simulink model "Complete_Model"  (R2023a)
settings: Solver=VariableStepAuto  StartTime=0.0  StopTime=22  MinStep=5e-5  RelTol=1e-3
values from neighbouring files (symbols this model uses):
  Cbus = 80e-3*n_wt_agg*u^2    (= 1.33333333333)    [param.m]
  D = 1e-3    (= 0.001)    [param.m]
  f = 50    [param.m]
  Fs = Vs * sqrt(2/3)/(2*pi*f)    [param.m]
  fsw = 4e3    (= 4000)    [param.m]
  Idr_rated = sqrt(2)*Is    [param.m]
  J = 127*n_wt_agg    (= 19050)    [param.m]
  ki_id = (wni^2)*Lr*sigma    (= 3.27733001332)    [param.m]
  ki_idg = (wnig^2)*Lg    (= 0.263189450696)    [param.m]
  ki_iq = ki_id    (= 3.27733001332)    [param.m]
  ki_iqg = ki_idg    (= 0.263189450696)    [param.m]
  ki_v = -300000*n_wt_agg    (= -45000000)    [param.m]
  Kopt = ((0.5*rho*pi*(Radius^5)*Cp_max)/(lambda_opt^3))    (= 296454.198186)    [param.m]
  kp_id = (2*wni*sigma*Lr) - Rr    (= 0.00384733333333)    [param.m]
  kp_idg = ((2*wnig*Lg)-Rg)    (= 0.00167538274858)    [param.m]
  kp_iq = kp_id    (= 0.00384733333333)    [param.m]
  kp_iqg = kp_idg    (= 0.00167538274858)    [param.m]
  kp_v = -1000*n_wt_agg    (= -150000)    [param.m]
  Kpg = 1/(1.5*Vr*sqrt(2/3))    [param.m]
  Kqg = -Kpg    [param.m]
  lambda_opt = 7.2    [param.m]
  Lg = 400e-6 /n_wt_agg    (= 2.66666666667e-06)    [param.m]
  Lm = 2.5e-3 /n_wt_agg    (= 1.66666666667e-05)    [param.m]
  Lr = Lm + Lsi    (= 1.72466666667e-05)    [param.m]
  Ls = Lm + Lsi    (= 1.72466666667e-05)    [param.m]
  Lsi = 0.087e-3 /n_wt_agg    (= 5.8e-07)    [param.m]
  N = 100    [param.m]
  n_wt_agg = 150    [param.m]
  p = 2    [param.m]
  Ps = 2e6*n_wt_agg    (= 300000000)    [param.m]
  Radius = 42    [param.m]
  Rg = 20e-6 /n_wt_agg    (= 1.33333333333e-07)    [param.m]
  rho = 1.225    [param.m]
  Rr = 2.9e-3 /n_wt_agg    (= 1.93333333333e-05)    [param.m]
  Rs = 2.6e-3 /n_wt_agg    (= 1.73333333333e-05)    [param.m]
  sigma = 1 - Lm^2 / (Ls*Lr)    (= 0.0661284179513)    [param.m]
  smax = 1/3    (= 0.333333333333)    [param.m]
  tab_lambda = [0.1:0.01:11.8]    [param.m]
  Vbus = 1150/u    (= 3450)    [param.m]
  Vs = 690    [param.m]
undefined symbols (not defined in the file or in its folder): Cp, D1, Delta, DiscIntNeverNeededParam, magnitude
libraries: spsSecondOrderFilterLib x133, spsThreePhaseVIMeasurementLib x58, spsPower3phInstantaneousLib x52, simulink x41, spsThreePhaseTransformerTwoWindingsLib x27, spsUniversalBridgeLib x22, spsPWMGenerator2LevelLib x22, spsSeriesRLCBranchLib x19, spsFirstOrderFilterLib x18, spsThreePhasePISectionLineLib x17, spsGroundLib x17, spsThreePhaseSeriesRLCBranchLib x17
(part 7 of 15)

== system Complete_Model/Equivalent Wind Park/300MW Eq WT10/Subsystem1 ==
  Constant4 : Constant  Value=0
  Step : Step  Time=50  After=-0.2*Ps  SampleTime=0
  Sum14 : Sum  Inputs=++|
  Out1 : Outport
  wires:
  Sum14/1 -> Out1/1
  Step/1 -> Sum14/1
  Constant4/1 -> Sum14/2

== system Complete_Model/Equivalent Wind Park/300MW Eq WT10/Subsystem2 ==
  Constant1 : Constant  Value=9
  Ramp1 : simulink/Sources/Ramp  slope=-0.4  start=13  InitialOutput=0
  Ramp2 : simulink/Sources/Ramp  slope=0.4  start=8  InitialOutput=0
  Sum1 : Sum  Inputs=++|
  Sum2 : Sum  Inputs=++|
  v_wind : Outport
  tag scope: v_wind3=global
  wires:
  Ramp1/1 -> Sum1/2
  Constant1/1 -> Sum2/2
  Ramp2/1 -> Sum1/1
  Sum1/1 -> Sum2/1
  Sum2/1 -> [v_wind3], v_wind/1

== system Complete_Model/Equivalent Wind Park/300MW Eq WT10/Subsystem8 ==
  Constant4 : Constant  Value=0
  Step : Step  Time=55  After=Idr_rated*0.2  SampleTime=0
  Sum14 : Sum  Inputs=++|
  Out1 : Outport
  wires:
  Sum14/1 -> Out1/1
  Constant4/1 -> Sum14/2
  Step/1 -> Sum14/1

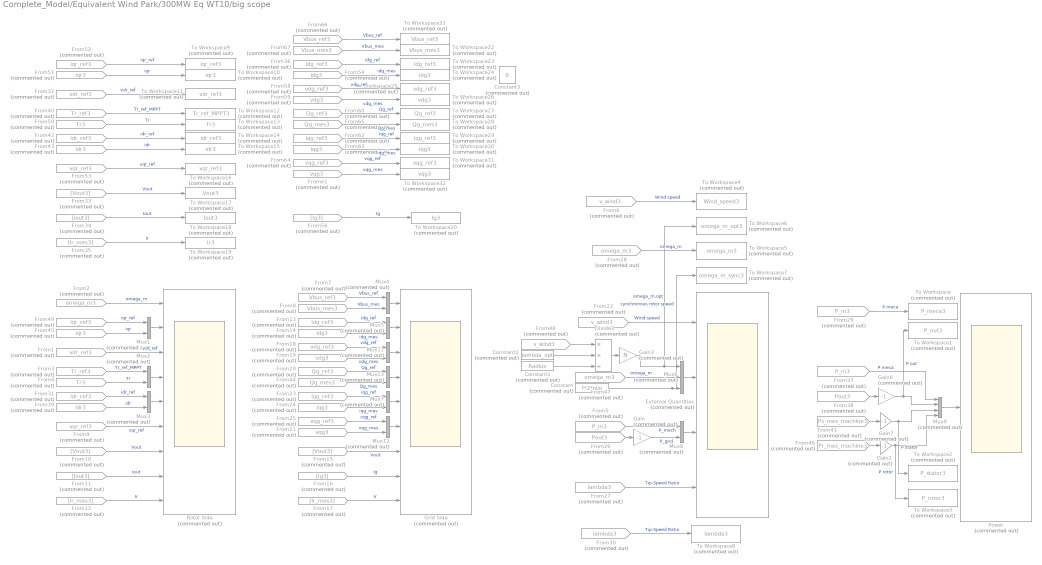
== system Complete_Model/Equivalent Wind Park/300MW Eq WT10/big scope ==
  Constant : Constant  Commented=on  Value=f*2*pi/p
  Constant1 : Constant  Commented=on  Value=Radius
  Constant2 : Constant  Commented=on  Value=lambda_opt
  Constant3 : Constant  Commented=on  Value=0
  Divide3 : Product  Commented=on  Inputs=**/
  External Quantities : Scope  Commented=on  NumInputPorts=4
  From1 : From  Commented=on  GotoTag=vdr_ref3  TagVisibility=global
  From10 : From  Commented=on  GotoTag=Vout3  TagVisibility=global
  From11 : From  Commented=on  GotoTag=Iout3  TagVisibility=global
  From12 : From  Commented=on  GotoTag=Ir_mes3  TagVisibility=global
  From13 : From  Commented=on  GotoTag=idg_ref3  TagVisibility=global
  From14 : From  Commented=on  GotoTag=idg3  TagVisibility=global
  From15 : From  Commented=on  GotoTag=Vout3  TagVisibility=global
  From16 : From  Commented=on  GotoTag=Ig3  TagVisibility=global
  From17 : From  Commented=on  GotoTag=Ir_mes3  TagVisibility=global
  From18 : From  Commented=on  GotoTag=vdg_ref3  TagVisibility=global
  From19 : From  Commented=on  GotoTag=vdg3  TagVisibility=global
  From2 : From  Commented=on  GotoTag=omega_m3  TagVisibility=global
  From20 : From  Commented=on  GotoTag=Qg_ref3  TagVisibility=global
  From21 : From  Commented=on  GotoTag=vqg3  TagVisibility=global
  From22 : From  Commented=on  GotoTag=v_wind3  TagVisibility=global
  From23 : From  Commented=on  GotoTag=iqg_ref3  TagVisibility=global
  From24 : From  Commented=on  GotoTag=iqg3  TagVisibility=global
  From25 : From  Commented=on  GotoTag=vqg_ref3  TagVisibility=global
  From26 : From  Commented=on  GotoTag=Pout3  TagVisibility=global
  From27 : From  Commented=on  GotoTag=lambda3  TagVisibility=global
  From28 : From  Commented=on  GotoTag=omega_m3  TagVisibility=global
  From29 : From  Commented=on  GotoTag=P_m3  TagVisibility=global
  From3 : From  Commented=on  GotoTag=Tr_ref3  TagVisibility=global
  From30 : From  Commented=on  GotoTag=lambda3  TagVisibility=global
  From31 : From  Commented=on  GotoTag=idr_ref3  TagVisibility=global
  From32 : From  Commented=on  GotoTag=vdr_ref3  TagVisibility=global
  From33 : From  Commented=on  GotoTag=Vout3  TagVisibility=global
  From34 : From  Commented=on  GotoTag=Iout3  TagVisibility=global
  From35 : From  Commented=on  GotoTag=Ir_mes3  TagVisibility=global
  From36 : From  Commented=on  GotoTag=idg_ref3  TagVisibility=global
  From37 : From  Commented=on  GotoTag=P_m3  TagVisibility=global
  From38 : From  Commented=on  GotoTag=Pout3  TagVisibility=global
  From39 : From  Commented=on  GotoTag=idr3  TagVisibility=global
  From4 : From  Commented=on  GotoTag=Tr3  TagVisibility=global
  From40 : From  Commented=on  GotoTag=Tr_ref3  TagVisibility=global
  From41 : From  Commented=on  GotoTag=Ps_mes_machine3  TagVisibility=global
  From42 : From  Commented=on  GotoTag=idr_ref3  TagVisibility=global
  From43 : From  Commented=on  GotoTag=idr3  TagVisibility=global
  From44 : From  Commented=on  GotoTag=Qg_mes3  TagVisibility=global
  From45 : From  Commented=on  GotoTag=iqr3  TagVisibility=global
  From46 : From  Commented=on  GotoTag=Pr_mes_machine3  TagVisibility=global
  From47 : From  Commented=on  GotoTag=omega_m3  TagVisibility=global
  From48 : From  Commented=on  GotoTag=v_wind3  TagVisibility=global
  From49 : From  Commented=on  GotoTag=iqr_ref3  TagVisibility=global
  From5 : From  Commented=on  GotoTag=P_m3  TagVisibility=global
  From50 : From  Commented=on  GotoTag=Tr3  TagVisibility=global
  From51 : From  Commented=on  GotoTag=iqr3  TagVisibility=global
  From52 : From  Commented=on  GotoTag=iqr_ref3  TagVisibility=global
  From53 : From  Commented=on  GotoTag=vqr_ref3  TagVisibility=global
  From54 : From  Commented=on  GotoTag=idg3  TagVisibility=global
  From56 : From  Commented=on  GotoTag=Ig3  TagVisibility=global
  From58 : From  Commented=on  GotoTag=vdg_ref3  TagVisibility=global
  From59 : From  Commented=on  GotoTag=vdg3  TagVisibility=global
  From6 : From  Commented=on  GotoTag=v_wind3  TagVisibility=global
  From60 : From  Commented=on  GotoTag=Qg_ref3  TagVisibility=global
  From61 : From  Commented=on  GotoTag=vqg3  TagVisibility=global
  From62 : From  Commented=on  GotoTag=iqg_ref3  TagVisibility=global
  From63 : From  Commented=on  GotoTag=iqg3  TagVisibility=global
  From64 : From  Commented=on  GotoTag=vqg_ref3  TagVisibility=global
  From65 : From  Commented=on  GotoTag=Qg_mes3  TagVisibility=global
  From66 : From  Commented=on  GotoTag=Vbus_ref3  TagVisibility=global
  From67 : From  Commented=on  GotoTag=Vbus_mes3  TagVisibility=global
  From7 : From  Commented=on  GotoTag=Vbus_ref3  TagVisibility=global
  From8 : From  Commented=on  GotoTag=Vbus_mes3  TagVisibility=global
  From9 : From  Commented=on  GotoTag=vqr_ref3  TagVisibility=global
  Gain{,2,6..7} (4 alike) : Gain  Commented=on  Gain=-1
  Gain3 : Gain  Commented=on  Gain=N
  Grid Side : Scope  Commented=on  NumInputPorts=9
  Mux{1..5,7..8,11..13} (10 alike) : Mux  Commented=on  Inputs=2
  Mux6 : Mux  Commented=on  Inputs=3
  Mux9 : Mux  Commented=on
  Power : Scope  Commented=on
  Rotor Side : Scope  Commented=on  NumInputPorts=9
  To Workspace : ToWorkspace  Commented=on  VariableName=P_meca3  SaveFormat=Timeseries  SampleTime=-1
  To Workspace1 : ToWorkspace  Commented=on  VariableName=P_out3  SaveFormat=Timeseries  SampleTime=-1
  To Workspace10 : ToWorkspace  Commented=on  VariableName=iqr3  SaveFormat=Timeseries  SampleTime=-1
  To Workspace11 : ToWorkspace  Commented=on  VariableName=vdr_ref3  SaveFormat=Timeseries  SampleTime=-1
  To Workspace12 : ToWorkspace  Commented=on  VariableName=Tr_ref_MPPT3  SaveFormat=Timeseries  SampleTime=-1
  To Workspace13 : ToWorkspace  Commented=on  VariableName=Tr3  SaveFormat=Timeseries  SampleTime=-1
  To Workspace14 : ToWorkspace  Commented=on  VariableName=idr_ref3  SaveFormat=Timeseries  SampleTime=-1
  To Workspace15 : ToWorkspace  Commented=on  VariableName=idr3  SaveFormat=Timeseries  SampleTime=-1
  To Workspace16 : ToWorkspace  Commented=on  VariableName=vqr_ref3  SaveFormat=Timeseries  SampleTime=-1
  To Workspace17 : ToWorkspace  Commented=on  VariableName=Vout3  SaveFormat=Timeseries  SampleTime=-1
  To Workspace18 : ToWorkspace  Commented=on  VariableName=Iout3  SaveFormat=Timeseries  SampleTime=-1
  To Workspace19 : ToWorkspace  Commented=on  VariableName=Ir3  SaveFormat=Timeseries  SampleTime=-1
  To Workspace2 : ToWorkspace  Commented=on  VariableName=P_stator3  SaveFormat=Timeseries  SampleTime=-1
  To Workspace20 : ToWorkspace  Commented=on  VariableName=Ig3  SaveFormat=Timeseries  SampleTime=-1
  To Workspace21 : ToWorkspace  Commented=on  VariableName=Vbus_ref3  SaveFormat=Timeseries  SampleTime=-1
  To Workspace22 : ToWorkspace  Commented=on  VariableName=Vbus_mes3  SaveFormat=Timeseries  SampleTime=-1
  To Workspace23 : ToWorkspace  Commented=on  VariableName=idg_ref3  SaveFormat=Timeseries  SampleTime=-1
  To Workspace24 : ToWorkspace  Commented=on  VariableName=idg3  SaveFormat=Timeseries  SampleTime=-1
  To Workspace25 : ToWorkspace  Commented=on  VariableName=vdg_ref3  SaveFormat=Timeseries  SampleTime=-1
  To Workspace26 : ToWorkspace  Commented=on  VariableName=vdg3  SaveFormat=Timeseries  SampleTime=-1
  To Workspace27 : ToWorkspace  Commented=on  VariableName=Qg_ref3  SaveFormat=Timeseries  SampleTime=-1
  To Workspace28 : ToWorkspace  Commented=on  VariableName=Qg_mes3  SaveFormat=Timeseries  SampleTime=-1
  To Workspace29 : ToWorkspace  Commented=on  VariableName=iqg_ref3  SaveFormat=Timeseries  SampleTime=-1
  To Workspace3 : ToWorkspace  Commented=on  VariableName=P_rotor3  SaveFormat=Timeseries  SampleTime=-1
  To Workspace30 : ToWorkspace  Commented=on  VariableName=iqg3  SaveFormat=Timeseries  SampleTime=-1
  To Workspace31 : ToWorkspace  Commented=on  VariableName=vqg_ref3  SaveFormat=Timeseries  SampleTime=-1
  To Workspace32 : ToWorkspace  Commented=on  VariableName=vqg3  SaveFormat=Timeseries  SampleTime=-1
  To Workspace4 : ToWorkspace  Commented=on  VariableName=Wind_speed3  SaveFormat=Timeseries  SampleTime=-1
  To Workspace5 : ToWorkspace  Commented=on  VariableName=omega_m3  SaveFormat=Timeseries  SampleTime=-1
  To Workspace6 : ToWorkspace  Commented=on  VariableName=omega_m_opt3  SaveFormat=Timeseries  SampleTime=-1
  To Workspace7 : ToWorkspace  Commented=on  VariableName=omega_m_sync3  SaveFormat=Timeseries  SampleTime=-1
  To Workspace8 : ToWorkspace  Commented=on  VariableName=lambda3  SaveFormat=Timeseries  SampleTime=-1
  To Workspace9 : ToWorkspace  Commented=on  VariableName=iqr_ref3  SaveFormat=Timeseries  SampleTime=-1
  wires:
  From61/1 -> To Workspace32/1  "vqg_mes"
  From64/1 -> To Workspace31/1  "vqg_ref"
  From63/1 -> To Workspace30/1  "iqg_mes"
  From62/1 -> To Workspace29/1  "iqg_ref"
  From65/1 -> To Workspace28/1  "Qg_mes"
  From59/1 -> To Workspace26/1  "vdg_mes"
  From60/1 -> To Workspace27/1  "Qg_ref"
  From58/1 -> To Workspace25/1  "vdg_ref"
  From54/1 -> To Workspace24/1  "idg_mes"
  From36/1 -> To Workspace23/1  "idg_ref"
  From67/1 -> To Workspace22/1  "Vbus_mes"
  From66/1 -> To Workspace21/1  "Vbus_ref"
  From56/1 -> To Workspace20/1  "Ig"
  From35/1 -> To Workspace19/1  "Ir"
  From34/1 -> To Workspace18/1  "Iout"
  From33/1 -> To Workspace17/1  "Vout"
  From53/1 -> To Workspace16/1  "vqr_ref"
  From43/1 -> To Workspace15/1  "idr"
  From42/1 -> To Workspace14/1  "idr_ref"
  From50/1 -> To Workspace13/1  "Tr"
  From40/1 -> To Workspace12/1  "Tr_ref_MPPT"
  From32/1 -> To Workspace11/1  "vdr_ref"
  From51/1 -> To Workspace10/1  "iqr"
  From52/1 -> To Workspace9/1  "iqr_ref"
  From30/1 -> To Workspace8/1  "Tip-Speed Ratio"
  From29/1 -> To Workspace/1  "P meca"
  From28/1 -> To Workspace5/1  "omega_m"
  From6/1 -> To Workspace4/1  "Wind speed"
  From27/1 -> External Quantities/4  "Tip-Speed Ratio"
  Gain3/1 -> Mux6/1, To Workspace6/1  "omega_m,opt"
  Divide3/1 -> Gain3/1
  Constant1/1 -> Divide3/3
  Constant2/1 -> Divide3/2
  From48/1 -> Divide3/1
  From39/1 -> Mux3/2  "idr"
  From4/1 -> Mux2/2  "Tr"
  From1/1 -> Rotor Side/3  "vdr_ref"
  From49/1 -> Mux1/1  "iqr_ref"
  Mux13/1 -> Grid Side/4
  From44/1 -> Mux13/2  "Qg_mes"
  From20/1 -> Mux13/1  "Qg_ref"
  Mux12/1 -> Grid Side/6
  From25/1 -> Mux12/1  "vqg_ref"
  From21/1 -> Mux12/2  "vqg_mes"
  Gain2/1 -> Mux9/4, To Workspace3/1  "P rotor"
  From38/1 -> Gain6/1
  Mux9/1 -> Power/1
  From37/1 -> Mux9/1  "P meca"
  From46/1 -> Gain2/1
  Gain7/1 -> Mux9/3, To Workspace2/1  "P stator"
  From41/1 -> Gain7/1
  Gain6/1 -> Mux9/2, To Workspace1/1  "P out"
  Mux11/1 -> Grid Side/3
  From19/1 -> Mux11/2  "vdg_mes"
  From18/1 -> Mux11/1  "vdg_ref"
  From5/1 -> Mux8/1  "P_mech"
  Constant/1 -> Mux6/3, To Workspace7/1  "synchronous rotor speed"
  From47/1 -> Mux6/2  "omega_m"
  From22/1 -> External Quantities/1  "Wind speed"
  Mux6/1 -> External Quantities/2
  Mux8/1 -> External Quantities/3
  From26/1 -> Gain/1
  Gain/1 -> Mux8/2  "P_grid"
  From2/1 -> Rotor Side/1  "omega_m"
  From13/1 -> Mux5/1  "idg_ref"
  Mux4/1 -> Grid Side/1
  From14/1 -> Mux5/2  "idg_mes"
  Mux5/1 -> Grid Side/2
  From8/1 -> Mux4/2  "Vbus_mes"
  Mux7/1 -> Grid Side/5
  From7/1 -> Mux4/1  "Vbus_ref"
  From24/1 -> Mux7/2  "iqg_mes"
  From17/1 -> Grid Side/9  "Ir"
  From15/1 -> Grid Side/7  "Vout"
  From23/1 -> Mux7/1  "iqg_ref"
  From16/1 -> Grid Side/8  "Ig"
  From12/1 -> Rotor Side/9  "Ir"
  From11/1 -> Rotor Side/8  "Iout"
  From10/1 -> Rotor Side/7  "Vout"
  From9/1 -> Rotor Side/6  "vqr_ref"
  From31/1 -> Mux3/1  "idr_ref"
  Mux3/1 -> Rotor Side/5
  Mux2/1 -> Rotor Side/4
  From3/1 -> Mux2/1  "Tr_ref_MPPT"
  Mux1/1 -> Rotor Side/2
  From45/1 -> Mux1/2  "iqr"

== system Complete_Model/Equivalent Wind Park/300MW Eq WT11/Aerodynamic Model ==
  note: Gearbox ratio
  note: Motor convention : Generated power
      |  is negative
  note: \Omega_m blades
  note: Cp
  note: \lambda = \Omega_m R / v_{wind}
  note: C_p (\lambda)
  note: v_{wind}
  Vw : Inport
  C_p (\lambda) : Lookup_n-D  NumberOfTableDimensions=1  BreakpointsForDimension1=tab_lambda  Table=Cp  InternalRulePriority=Speed  RndMeth=Simplest
  Constant : Constant  Value=Radius
  Divide : Product  Inputs=*/
  Divide1 : Product  Inputs=**/
  Fcn2 : Fcn  Expr=0.5*rho*pi*Radius^2*u(2)^3*u(1)
  Gain : Gain  Gain=1/N
  Gain2 : Gain  Gain=n_wt_agg
  Gain4 : Gain  Gain=-1
  Mux2 : Mux  Inputs=2
  Tm : Outport
  tag scope: P_m22=global, Tm22=global, lambda22=global
  wires:
  Gain4/1 -> Divide/1
  Constant/1 -> Divide1/1
  Divide/1 -> Tm/1, [Tm22]
  [omega_m22] -> Divide/2
  Gain2/1 -> Gain4/1, [P_m22]
  Fcn2/1 -> Gain2/1
  Mux2/1 -> Fcn2/1
  C_p (\lambda)/1 -> Mux2/1
  Divide1/1 -> [lambda22], C_p (\lambda)/1  "lambda"
  Vw/1 -> Mux2/2, Divide1/3
  Gain/1 -> Divide1/2
  [omega_m22] -> Gain/1

== system Complete_Model/Equivalent Wind Park/300MW Eq WT11/Grid Side Control ==
  Vbus_ref : Inport
  Qg_ref : Inport  Port=2
  Vbus : Inport  Port=3
  Ig_mes : Inport  Port=4
  Vs_mes : Inport  Port=5
  Demux : Demux  Outputs=2
  Demux1 : Demux  Outputs=2
  Gain : Gain  Gain=Kqg
  Gain1 : Gain  Gain=Kpg
  Normalized for PWM : Gain  Gain=1/(Vbus/2)
  "PI " : Subsystem
  PI id : Subsystem
  PI iq : Subsystem
  Sum : Sum  Inputs=|++
  Vs_mes1 : InportShadow  Port=5
  abc->alpha/beta : Subsystem
  abc->alpha/beta1 : Subsystem
  alpha/beta->abc : Subsystem
  alpha/beta->d/q : Subsystem
  alpha/beta->d/q1 : Subsystem
  angle2 : Subsystem
  cancellation of coupling terms : Subsystem
  d/q->alpha/beta : Subsystem
  Vabc_ref : Outport
  tag scope: Qg_ref22=global, Vbus_ref22=global, idg22=global, idg_ref22=global, iqg22=global, iqg_ref22=global, vdg22=global, vqg22=global
  wires:
  Vs_mes1/1 -> Sum/2
  angle2/1(theta_g) -> [theta_grid22]
  [theta_grid22] -> alpha/beta->d/q1/2(theta)
  [theta_grid22] -> d/q->alpha/beta/2(theta)
  [theta_grid22] -> alpha/beta->d/q/2(theta)
  Demux/2 -> PI iq/2(meas), [iqg22]
  Demux/1 -> PI id/2(meas), [idg22]
  Vbus/1 -> "PI "/2(meas)
  Demux1/1 -> [vdg22]
  Demux1/2 -> [vqg22]
  alpha/beta->d/q1/1(d/q) -> Demux1/1
  abc->alpha/beta1/1(alpha/beta) -> alpha/beta->d/q1/1(D/Q)
  [Vg22] -> abc->alpha/beta1/1(abc)
  cancellation of coupling terms/1(out) -> d/q->alpha/beta/1(d/q)
  Sum/1 -> Normalized for PWM/1
  alpha/beta->abc/1(abc) -> Sum/1
  Qg_ref/1 -> Gain/1, [Qg_ref22]
  Gain1/1 -> PI id/1(ref), [idg_ref22]  "idg"
  "PI "/1(out) -> Gain1/1  "Pg_ref"
  Gain/1 -> [iqg_ref22], PI iq/1(ref)  "iqg"
  Vbus_ref/1 -> "PI "/1(ref), [Vbus_ref22]
  alpha/beta->d/q/1(d/q) -> Demux/1
  PI iq/1(out) -> cancellation of coupling terms/2(Vq*)
  PI id/1(out) -> cancellation of coupling terms/1(Vd*)
  Vs_mes/1 -> angle2/1(Vs_mes)
  Normalized for PWM/1 -> Vabc_ref/1
  abc->alpha/beta/1(alpha/beta) -> alpha/beta->d/q/1(D/Q)
  Ig_mes/1 -> abc->alpha/beta/1(abc)
  d/q->alpha/beta/1(D/Q) -> alpha/beta->abc/1(D/Q)

== system Complete_Model/Equivalent Wind Park/300MW Eq WT11/Power Calculations ==
  Demux{,1..3} (4 alike) : Demux  Outputs=2
  Divide : Product  Inputs=**
  Gain : Gain  Gain=3/2
  Gain1 : Gain  Gain=-1
  Gain2 : Gain  Gain=-1
  Power (3ph, Instantaneous){1..4} (4 alike) : spsPower3phInstantaneousLib/Power (3ph, Instantaneous)
  Scope{1..8,10..13} (12 alike) : Scope
  Scope9 : Scope  NumInputPorts=2
  "Second-Order " : spsSecondOrderFilterLib/Second-Order Filter  FilterType=Lowpass  Fo=17  Zeta=0.7  Ts=7.4074e-05  Initialize=off  Vac_Init="[0,  0,  60]"  Vdc_Init=0  PlotResponse=off  FreqRange=[0, 500, 1]
      settings: AttributesFormatString=f0=%<Fo>
  Second-Order 1 : spsSecondOrderFilterLib/Second-Order Filter  FilterType=Lowpass  Fo=17  Zeta=0.7  Ts=7.4074e-05  Initialize=off  Vac_Init="[0,  0,  60]"  Vdc_Init=0  PlotResponse=off  FreqRange=[0, 500, 1]
      settings: AttributesFormatString=f0=%<Fo>
  Second-Order Low-Pass Filter {1..7} (7 alike) : spsSecondOrderFilterLib/Second-Order Filter  FilterType=Lowpass  Fo=17  Zeta=0.7  Ts=7.4074e-05  Initialize=off  Vac_Init="[0,  0,  60]"  Vdc_Init=0  PlotResponse=off  FreqRange=[0, 500, 1]
      settings: AttributesFormatString=f0=%<Fo>
  Subsystem : Subsystem
  Subsystem1 : Subsystem
  Subsystem2 : Subsystem
  Subsystem3 : Subsystem
  To Workspace1 : ToWorkspace  VariableName=Iout_d22  SaveFormat=Timeseries  SampleTime=-1
  To Workspace2 : ToWorkspace  VariableName=Ig_d22  SaveFormat=Timeseries  SampleTime=-1
  To Workspace21 : ToWorkspace  VariableName=Vout_d22  SaveFormat=Timeseries  SampleTime=-1
  To Workspace3 : ToWorkspace  VariableName=Vg_d22  SaveFormat=Timeseries  SampleTime=-1
  ToFile_Ig_d22 : ToFile  Filename=Ig_d22.mat  SaveFormat=Timeseries
  ToFile_Iout_d22 : ToFile  Filename=Iout_d22.mat  SaveFormat=Timeseries
  ToFile_Vg_d22 : ToFile  Filename=Vg_d22.mat  SaveFormat=Timeseries
  ToFile_Vout_d22 : ToFile  Filename=Vout_d22.mat  SaveFormat=Timeseries
  alpha/beta->d/q : Subsystem
  alpha/beta->d/q1 : Subsystem
  alpha/beta->d/q2 : Subsystem
  alpha/beta->d/q3 : Subsystem
  angle1 : Subsystem
  angle2 : Subsystem
  tag scope: Pg_mes22=global, Pout22=global, Pr_mes_machine22=global, Ps_mes_machine22=global, Qg_mes22=global, Qout22=global, Qr_mes_machine22=global, Qs_mes_machine22=global
  wires:
  [Vg22] -> angle1/1(Vs_mes), Subsystem2/1(abc)
  Subsystem2/1(alpha/beta) -> alpha/beta->d/q2/1(D/Q)
  alpha/beta->d/q2/1(d/q) -> Demux2/1
  Demux2/1 -> ToFile_Vg_d22/1, Scope10/1, To Workspace3/1  "Vg_d22"
  Demux2/2 -> Scope11/1
  Demux3/2 -> Scope13/1
  Demux3/1 -> ToFile_Ig_d22/1, Scope12/1, To Workspace2/1  "Ig_d22"
  alpha/beta->d/q3/1(d/q) -> Demux3/1
  Subsystem3/1(alpha/beta) -> alpha/beta->d/q3/1(D/Q)
  angle1/1(theta_g) -> alpha/beta->d/q3/2(theta), alpha/beta->d/q2/2(theta)
  [Ig22] -> Subsystem3/1(abc)
  Gain2/1 -> Second-Order 1/1
  Gain/1 -> Gain2/1
  Gain1/1 -> Scope9/2
  Second-Order 1/1 -> Scope9/1  "Pout"
  Divide/1 -> Gain/1
  [Iout22] -> Subsystem1/1(abc)
  angle2/1(theta_g) -> alpha/beta->d/q/2(theta), alpha/beta->d/q1/2(theta)
  Subsystem1/1(alpha/beta) -> alpha/beta->d/q1/1(D/Q)
  alpha/beta->d/q1/1(d/q) -> Demux1/1
  Demux1/1 -> ToFile_Iout_d22/1, Scope7/1, Divide/2, To Workspace1/1  "Iout_d22"
  Demux1/2 -> Scope8/1
  Demux/2 -> Scope6/1
  Demux/1 -> ToFile_Vout_d22/1, Scope2/1, To Workspace21/1, Divide/1  "Vout_d22"
  alpha/beta->d/q/1(d/q) -> Demux/1
  Subsystem/1(alpha/beta) -> alpha/beta->d/q/1(D/Q)
  [Vout22] -> Subsystem/1(abc), angle2/1(Vs_mes)
  [Vr_mes22] -> "Power (3ph, Instantaneous)4"/1
  "Power (3ph, Instantaneous)1"/1 -> Second-Order Low-Pass Filter 7/1  "Pr"
  "Power (3ph, Instantaneous)4"/1 -> Second-Order Low-Pass Filter 5/1  "Pr"
  [Vout22] -> "Power (3ph, Instantaneous)2"/1
  "Power (3ph, Instantaneous)3"/2 -> Second-Order Low-Pass Filter 2/1
  [Vg22] -> "Power (3ph, Instantaneous)1"/1
  Second-Order Low-Pass Filter 2/1 -> [Qs_mes_machine22]  "Q_stator"
  [Vs_mes_machine22] -> "Power (3ph, Instantaneous)3"/1
  "Power (3ph, Instantaneous)2"/1 -> "Second-Order "/1
  Second-Order Low-Pass Filter 5/1 -> [Pr_mes_machine22]  "P_rotor"
  [Is_mes_machine22] -> "Power (3ph, Instantaneous)3"/2, Scope4/1
  Second-Order Low-Pass Filter 7/1 -> [Pg_mes22]  "P_rotor"
  [Ir_mes22] -> "Power (3ph, Instantaneous)4"/2, Scope5/1
  "Second-Order "/1 -> [Pout22], Gain1/1  "Pout"
  Second-Order Low-Pass Filter 6/1 -> [Qg_mes22]  "Q_rotor"
  Second-Order Low-Pass Filter 4/1 -> [Qr_mes_machine22]  "Q_rotor"
  "Power (3ph, Instantaneous)3"/1 -> Second-Order Low-Pass Filter 3/1  "Ps"
  [Ig22] -> Scope1/1, "Power (3ph, Instantaneous)1"/2
  "Power (3ph, Instantaneous)4"/2 -> Second-Order Low-Pass Filter 4/1
  [Iout22] -> "Power (3ph, Instantaneous)2"/2, Scope3/1
  "Power (3ph, Instantaneous)1"/2 -> Second-Order Low-Pass Filter 6/1
  Second-Order Low-Pass Filter 3/1 -> [Ps_mes_machine22]  "P_stator"
  Second-Order Low-Pass Filter 1/1 -> [Qout22]  "Qout"
  "Power (3ph, Instantaneous)2"/2 -> Second-Order Low-Pass Filter 1/1

== system Complete_Model/Equivalent Wind Park/300MW Eq WT11/Rotor Side Control ==
  note: Stator referred
  idr_ref : Inport
  Ir_mes : Inport  Port=2
  Vs_mes : Inport  Port=3
  theta_m_mes : Inport  Port=4
  speed_mes : Inport  Port=5
  D/Q->abc : Subsystem
  D/Q->d/q : Subsystem
  Demux : Demux  Outputs=2
  Gain : Gain  Gain=1/(-1.5*p*(Lm/Ls)*Fs)
  Gain1 : Gain  Gain=1/u
  Gain2 : Gain  Gain=1/u
  MPPT : Subsystem
  Normalized for PWM : Gain  Gain=1/(Vbus/2)
  PI id : Subsystem
  PI iq : Subsystem
  abc->alpha/beta : Subsystem
  angle : Subsystem
  cancellation of coupling terms : Subsystem
  d/q->D/Q : Subsystem
  Vabc_ref : Outport
  tag scope: Tr_ref22=global, idr22=global, idr_ref22=global, iqr22=global, iqr_ref22=global, vrabc_ref22=global
  wires:
  MPPT/1(Tm,opt) -> Gain/1, [Tr_ref22]
  d/q->D/Q/1(D/Q) -> D/Q->abc/1(D/Q)
  abc->alpha/beta/1(alpha/beta) -> D/Q->d/q/1(D/Q)
  Gain2/1 -> [vrabc_ref22], Normalized for PWM/1  "Rotor referred"
  D/Q->abc/1(abc) -> Gain2/1
  Normalized for PWM/1 -> Vabc_ref/1
  Gain1/1 -> abc->alpha/beta/1(abc)
  idr_ref/1 -> PI id/1(ref), [idr_ref22]
  Gain/1 -> PI iq/1(ref), [iqr_ref22]
  Demux/2 -> PI iq/2(meas), [iqr22]
  Demux/1 -> [idr22], PI id/2(meas)
  D/Q->d/q/1(d/q) -> Demux/1  "i_dq = rotor ref frame"
  speed_mes/1 -> MPPT/1(omega_m), cancellation of coupling terms/3(omega_m)
  cancellation of coupling terms/1(out) -> d/q->D/Q/1(d/q)  "Vdr_ref,Vqr_ref"
  PI iq/1(out) -> cancellation of coupling terms/2(Vq*)
  PI id/1(out) -> cancellation of coupling terms/1(Vd*)
  angle/1(theta_r) -> D/Q->d/q/2(theta), d/q->D/Q/2(theta)
  Vs_mes/1 -> angle/1(Vs_mes)
  theta_m_mes/1 -> angle/2(theta_m)
  Ir_mes/1 -> Gain1/1

== system Complete_Model/Equivalent Wind Park/300MW Eq WT11/Subsystem ==
  In1 : Inport
  Bus Selector : BusSelector  OutputSignals=Mechanical.Rotor angle thetam (rad),Mechanical.Rotor speed (wm),Mechanical.Electromagnetic torque Te (N*m)
  Second-Order Low-Pass Filter 3 : spsSecondOrderFilterLib/Second-Order Filter  FilterType=Lowpass  Fo=17  Zeta=0.7  Ts=7.4074e-05  Initialize=off  Vac_Init="[0,  0,  60]"  Vdc_Init=0  PlotResponse=off  FreqRange=[0, 500, 1]
      settings: AttributesFormatString=f0=%<Fo>
  tag scope: Tr22=global, omega_m22=global, theta22=global
  wires:
  Second-Order Low-Pass Filter 3/1 -> [omega_m22]
  Bus Selector/3 -> [Tr22]  "<Electromagnetic torque Te (N*m)>"
  Bus Selector/1 -> [theta22]  "<Rotor angle thetam (rad)>"
  In1/1 -> Bus Selector/1
  Bus Selector/2 -> Second-Order Low-Pass Filter 3/1  "<Rotor speed (wm)>"

== system Complete_Model/Equivalent Wind Park/300MW Eq WT11/Subsystem1 ==
  Constant4 : Constant  Value=0
  Step : Step  Time=50  After=-0.2*Ps  SampleTime=0
  Sum14 : Sum  Inputs=++|
  Out1 : Outport
  wires:
  Sum14/1 -> Out1/1
  Step/1 -> Sum14/1
  Constant4/1 -> Sum14/2

== system Complete_Model/Equivalent Wind Park/300MW Eq WT11/Subsystem2 ==
  Constant1 : Constant  Value=9
  Constant3 : Constant  Commented=on  Value=10
  Ramp1 : simulink/Sources/Ramp  slope=-0.4  start=13  InitialOutput=0
  Ramp2 : simulink/Sources/Ramp  slope=0.4  start=8  InitialOutput=0
  Ramp5 : simulink/Sources/Ramp  Commented=on  slope=-1  start=11  InitialOutput=0
  Ramp6 : simulink/Sources/Ramp  Commented=on  slope=1  start=10  InitialOutput=0
  Sum1 : Sum  Inputs=++|
  Sum2 : Sum  Inputs=++|
  Sum5 : Sum  Commented=on  Inputs=++|
  Sum6 : Sum  Commented=on  Inputs=++|
  v_wind : Outport
  tag scope: v_wind22=global
  wires:
  Sum5/1 -> Sum6/1
  Constant3/1 -> Sum6/2
  Ramp6/1 -> Sum5/1
  Ramp5/1 -> Sum5/2
  Ramp1/1 -> Sum1/2
  Constant1/1 -> Sum2/2
  Ramp2/1 -> Sum1/1
  Sum1/1 -> Sum2/1
  Sum2/1 -> v_wind/1, [v_wind22]

== system Complete_Model/Equivalent Wind Park/300MW Eq WT11/Subsystem8 ==
  Constant4 : Constant  Value=0
  Step : Step  Time=55  After=Idr_rated*0.2  SampleTime=0
  Sum14 : Sum  Inputs=++|
  Out1 : Outport
  wires:
  Sum14/1 -> Out1/1
  Constant4/1 -> Sum14/2
  Step/1 -> Sum14/1

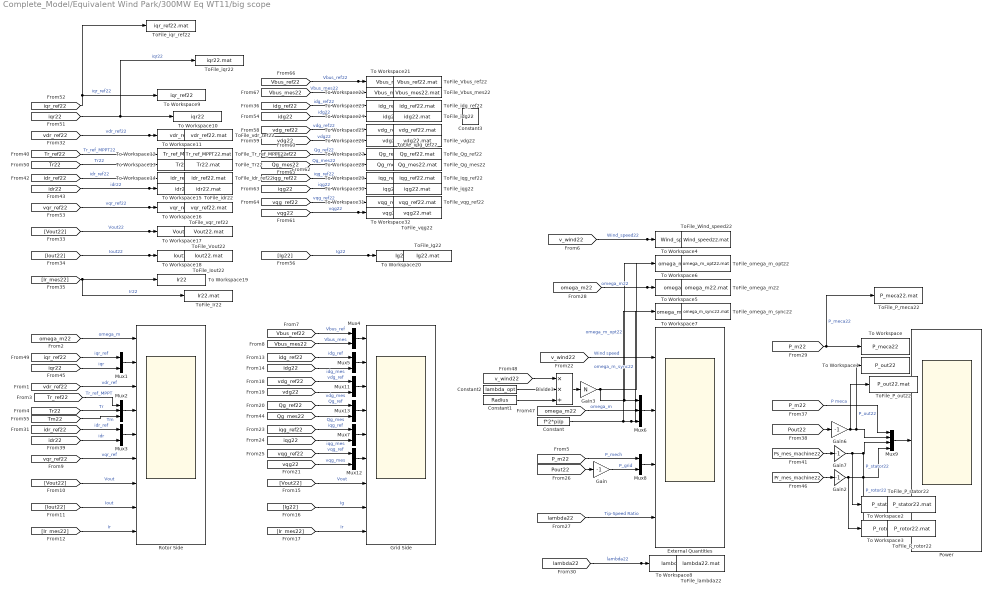
== system Complete_Model/Equivalent Wind Park/300MW Eq WT11/big scope ==
  Constant : Constant  Value=f*2*pi/p
  Constant1 : Constant  Value=Radius
  Constant2 : Constant  Value=lambda_opt
  Constant3 : Constant  Value=0
  Divide3 : Product  Inputs=**/
  External Quantities : Scope  NumInputPorts=4
  Gain{,2,6..7} (4 alike) : Gain  Gain=-1
  Gain3 : Gain  Gain=N
  Grid Side : Scope  NumInputPorts=9
  Mux{1,3..5,7..8,11..13} (9 alike) : Mux  Inputs=2
  Mux2 : Mux  Inputs=3
  Mux6 : Mux  Inputs=3
  Mux9 : Mux
  Power : Scope
  Rotor Side : Scope  NumInputPorts=9
  To Workspace : ToWorkspace  VariableName=P_meca22  SaveFormat=Timeseries  SampleTime=-1
  To Workspace1 : ToWorkspace  VariableName=P_out22  SaveFormat=Timeseries  SampleTime=-1
  To Workspace10 : ToWorkspace  VariableName=iqr22  SaveFormat=Timeseries  SampleTime=-1
  To Workspace11 : ToWorkspace  VariableName=vdr_ref22  SaveFormat=Timeseries  SampleTime=-1
  To Workspace12 : ToWorkspace  VariableName=Tr_ref_MPPT22  SaveFormat=Timeseries  SampleTime=-1
  To Workspace13 : ToWorkspace  VariableName=Tr22  SaveFormat=Timeseries  SampleTime=-1
  To Workspace14 : ToWorkspace  VariableName=idr_ref22  SaveFormat=Timeseries  SampleTime=-1
  To Workspace15 : ToWorkspace  VariableName=idr22  SaveFormat=Timeseries  SampleTime=-1
  To Workspace16 : ToWorkspace  VariableName=vqr_ref22  SaveFormat=Timeseries  SampleTime=-1
  To Workspace17 : ToWorkspace  VariableName=Vout22  SaveFormat=Timeseries  SampleTime=-1
  To Workspace18 : ToWorkspace  VariableName=Iout22  SaveFormat=Timeseries  SampleTime=-1
  To Workspace19 : ToWorkspace  VariableName=Ir22  SaveFormat=Timeseries  SampleTime=-1
  To Workspace2 : ToWorkspace  VariableName=P_stator22  SaveFormat=Timeseries  SampleTime=-1
  To Workspace20 : ToWorkspace  VariableName=Ig22  SaveFormat=Timeseries  SampleTime=-1
  To Workspace21 : ToWorkspace  VariableName=Vbus_ref22  SaveFormat=Timeseries  SampleTime=-1
  To Workspace22 : ToWorkspace  VariableName=Vbus_mes22  SaveFormat=Timeseries  SampleTime=-1
  To Workspace23 : ToWorkspace  VariableName=idg_ref22  SaveFormat=Timeseries  SampleTime=-1
  To Workspace24 : ToWorkspace  VariableName=idg22  SaveFormat=Timeseries  SampleTime=-1
  To Workspace25 : ToWorkspace  VariableName=vdg_ref22  SaveFormat=Timeseries  SampleTime=-1
  To Workspace26 : ToWorkspace  VariableName=vdg22  SaveFormat=Timeseries  SampleTime=-1
  To Workspace27 : ToWorkspace  VariableName=Qg_ref22  SaveFormat=Timeseries  SampleTime=-1
  To Workspace28 : ToWorkspace  VariableName=Qg_mes22  SaveFormat=Timeseries  SampleTime=-1
  To Workspace29 : ToWorkspace  VariableName=iqg_ref22  SaveFormat=Timeseries  SampleTime=-1
  To Workspace3 : ToWorkspace  VariableName=P_rotor22  SaveFormat=Timeseries  SampleTime=-1
  To Workspace30 : ToWorkspace  VariableName=iqg22  SaveFormat=Timeseries  SampleTime=-1
  To Workspace31 : ToWorkspace  VariableName=vqg_ref22  SaveFormat=Timeseries  SampleTime=-1
  To Workspace32 : ToWorkspace  VariableName=vqg22  SaveFormat=Timeseries  SampleTime=-1
  To Workspace4 : ToWorkspace  VariableName=Wind_speed22  SaveFormat=Timeseries  SampleTime=-1
  To Workspace5 : ToWorkspace  VariableName=omega_m22  SaveFormat=Timeseries  SampleTime=-1
  To Workspace6 : ToWorkspace  VariableName=omega_m_opt22  SaveFormat=Timeseries  SampleTime=-1
  To Workspace7 : ToWorkspace  VariableName=omega_m_sync22  SaveFormat=Timeseries  SampleTime=-1
  To Workspace8 : ToWorkspace  VariableName=lambda22  SaveFormat=Timeseries  SampleTime=-1
  To Workspace9 : ToWorkspace  VariableName=iqr_ref22  SaveFormat=Timeseries  SampleTime=-1
  ToFile_Ig22 : ToFile  Filename=Ig22.mat  SaveFormat=Timeseries
  ToFile_Iout22 : ToFile  Filename=Iout22.mat  SaveFormat=Timeseries
  ToFile_Ir22 : ToFile  Filename=Ir22.mat  SaveFormat=Timeseries
  ToFile_P_meca22 : ToFile  Filename=P_meca22.mat  SaveFormat=Timeseries
  ToFile_P_out22 : ToFile  Filename=P_out22.mat  SaveFormat=Timeseries
  ToFile_P_rotor22 : ToFile  Filename=P_rotor22.mat  SaveFormat=Timeseries
  ToFile_P_stator22 : ToFile  Filename=P_stator22.mat  SaveFormat=Timeseries
  ToFile_Qg_mes22 : ToFile  Filename=Qg_mes22.mat  SaveFormat=Timeseries
  ToFile_Qg_ref22 : ToFile  Filename=Qg_ref22.mat  SaveFormat=Timeseries
  ToFile_Tr22 : ToFile  Filename=Tr22.mat  SaveFormat=Timeseries
  ToFile_Tr_ref_MPPT22 : ToFile  Filename=Tr_ref_MPPT22.mat  SaveFormat=Timeseries
  ToFile_Vbus_mes22 : ToFile  Filename=Vbus_mes22.mat  SaveFormat=Timeseries
  ToFile_Vbus_ref22 : ToFile  Filename=Vbus_ref22.mat  SaveFormat=Timeseries
  ToFile_Vout22 : ToFile  Filename=Vout22.mat  SaveFormat=Timeseries
  ToFile_Wind_speed22 : ToFile  Filename=Wind_speed22.mat  SaveFormat=Timeseries
  ToFile_idg22 : ToFile  Filename=idg22.mat  SaveFormat=Timeseries
  ToFile_idg_ref22 : ToFile  Filename=idg_ref22.mat  SaveFormat=Timeseries
  ToFile_idr22 : ToFile  Filename=idr22.mat  SaveFormat=Timeseries
  ToFile_idr_ref22 : ToFile  Filename=idr_ref22.mat  SaveFormat=Timeseries
  ToFile_iqg22 : ToFile  Filename=iqg22.mat  SaveFormat=Timeseries
  ToFile_iqg_ref22 : ToFile  Filename=iqg_ref22.mat  SaveFormat=Timeseries
  ToFile_iqr22 : ToFile  Filename=iqr22.mat  SaveFormat=Timeseries
  ToFile_iqr_ref22 : ToFile  Filename=iqr_ref22.mat  SaveFormat=Timeseries
  ToFile_lambda22 : ToFile  Filename=lambda22.mat  SaveFormat=Timeseries
  ToFile_omega_m22 : ToFile  Filename=omega_m22.mat  SaveFormat=Timeseries
  ToFile_omega_m_opt22 : ToFile  Filename=omega_m_opt22.mat  SaveFormat=Timeseries
  ToFile_omega_m_sync22 : ToFile  Filename=omega_m_sync22.mat  SaveFormat=Timeseries
  ToFile_vdg22 : ToFile  Filename=vdg22.mat  SaveFormat=Timeseries
  ToFile_vdg_ref22 : ToFile  Filename=vdg_ref22.mat  SaveFormat=Timeseries
  ToFile_vdr_ref22 : ToFile  Filename=vdr_ref22.mat  SaveFormat=Timeseries
  ToFile_vqg22 : ToFile  Filename=vqg22.mat  SaveFormat=Timeseries
  ToFile_vqg_ref22 : ToFile  Filename=vqg_ref22.mat  SaveFormat=Timeseries
  ToFile_vqr_ref22 : ToFile  Filename=vqr_ref22.mat  SaveFormat=Timeseries
  wires:
  [vqg22] -> To Workspace32/1, ToFile_vqg22/1  "vqg22"
  [vqg_ref22] -> To Workspace31/1, ToFile_vqg_ref22/1  "vqg_ref22"
  [iqg22] -> To Workspace30/1, ToFile_iqg22/1  "iqg22"
  [iqg_ref22] -> To Workspace29/1, ToFile_iqg_ref22/1  "iqg_ref22"
  [Qg_mes22] -> To Workspace28/1, ToFile_Qg_mes22/1  "Qg_mes22"
  [vdg22] -> To Workspace26/1, ToFile_vdg22/1  "vdg22"
  [Qg_ref22] -> To Workspace27/1, ToFile_Qg_ref22/1  "Qg_ref22"
  [vdg_ref22] -> To Workspace25/1, ToFile_vdg_ref22/1  "vdg_ref22"
  [idg22] -> To Workspace24/1, ToFile_idg22/1  "idg22"
  [idg_ref22] -> To Workspace23/1, ToFile_idg_ref22/1  "idg_ref22"
  [Vbus_mes22] -> To Workspace22/1, ToFile_Vbus_mes22/1  "Vbus_mes22"
  [Vbus_ref22] -> To Workspace21/1, ToFile_Vbus_ref22/1  "Vbus_ref22"
  [Ig22] -> To Workspace20/1, ToFile_Ig22/1  "Ig22"
  [Ir_mes22] -> To Workspace19/1, ToFile_Ir22/1  "Ir22"
  [Iout22] -> To Workspace18/1, ToFile_Iout22/1  "Iout22"
  [Vout22] -> To Workspace17/1, ToFile_Vout22/1  "Vout22"
  [vqr_ref22] -> To Workspace16/1, ToFile_vqr_ref22/1  "vqr_ref22"
  [idr22] -> To Workspace15/1, ToFile_idr22/1  "idr22"
  [idr_ref22] -> To Workspace14/1, ToFile_idr_ref22/1  "idr_ref22"
  [Tr22] -> To Workspace13/1, ToFile_Tr22/1  "Tr22"
  [Tr_ref22] -> To Workspace12/1, ToFile_Tr_ref_MPPT22/1  "Tr_ref_MPPT22"
  [vdr_ref22] -> To Workspace11/1, ToFile_vdr_ref22/1  "vdr_ref22"
  [iqr22] -> To Workspace10/1, ToFile_iqr22/1  "iqr22"
  [iqr_ref22] -> To Workspace9/1, ToFile_iqr_ref22/1  "iqr_ref22"
  [lambda22] -> To Workspace8/1, ToFile_lambda22/1  "lambda22"
  [P_m22] -> To Workspace/1, ToFile_P_meca22/1  "P_meca22"
  [omega_m22] -> To Workspace5/1, ToFile_omega_m22/1  "omega_m22"
  [v_wind22] -> To Workspace4/1, ToFile_Wind_speed22/1  "Wind_speed22"
  [lambda22] -> External Quantities/4  "Tip-Speed Ratio"
  Gain3/1 -> Mux6/1, To Workspace6/1, ToFile_omega_m_opt22/1  "omega_m_opt22"
  Divide3/1 -> Gain3/1
  Constant1/1 -> Divide3/3
  Constant2/1 -> Divide3/2
  [v_wind22] -> Divide3/1
  [idr22] -> Mux3/2  "idr"
  [Tr22] -> Mux2/2  "Tr"
  [vdr_ref22] -> Rotor Side/3  "vdr_ref"
  [iqr_ref22] -> Mux1/1  "iqr_ref"
  Mux13/1 -> Grid Side/4
  [Qg_mes22] -> Mux13/2  "Qg_mes"
  [Qg_ref22] -> Mux13/1  "Qg_ref"
  Mux12/1 -> Grid Side/6
  [vqg_ref22] -> Mux12/1  "vqg_ref"
  [vqg22] -> Mux12/2  "vqg_mes"
  Gain2/1 -> ToFile_P_rotor22/1, Mux9/4, To Workspace3/1  "P_rotor22"
  [Pout22] -> Gain6/1
  Mux9/1 -> Power/1
  [P_m22] -> Mux9/1  "P meca"
  [Pr_mes_machine22] -> Gain2/1
  Gain7/1 -> ToFile_P_stator22/1, Mux9/3, To Workspace2/1  "P_stator22"
  [Ps_mes_machine22] -> Gain7/1
  Gain6/1 -> Mux9/2, To Workspace1/1, ToFile_P_out22/1  "P_out22"
  Mux11/1 -> Grid Side/3
  [vdg22] -> Mux11/2  "vdg_mes"
  [vdg_ref22] -> Mux11/1  "vdg_ref"
  [P_m22] -> Mux8/1  "P_mech"
  Constant/1 -> Mux6/3, To Workspace7/1, ToFile_omega_m_sync22/1  "omega_m_sync22"
  [omega_m22] -> Mux6/2  "omega_m"
  [v_wind22] -> External Quantities/1  "Wind speed"
  Mux6/1 -> External Quantities/2
  Mux8/1 -> External Quantities/3
  [Pout22] -> Gain/1
  Gain/1 -> Mux8/2  "P_grid"
  [omega_m22] -> Rotor Side/1  "omega_m"
  [idg_ref22] -> Mux5/1  "idg_ref"
  Mux4/1 -> Grid Side/1
  [idg22] -> Mux5/2  "idg_mes"
  Mux5/1 -> Grid Side/2
  [Vbus_mes22] -> Mux4/2  "Vbus_mes"
  Mux7/1 -> Grid Side/5
  [Vbus_ref22] -> Mux4/1  "Vbus_ref"
  [iqg22] -> Mux7/2  "iqg_mes"
  [Ir_mes22] -> Grid Side/9  "Ir"
  [Vout22] -> Grid Side/7  "Vout"
  [iqg_ref22] -> Mux7/1  "iqg_ref"
  [Ig22] -> Grid Side/8  "Ig"
  [Ir_mes22] -> Rotor Side/9  "Ir"
  [Iout22] -> Rotor Side/8  "Iout"
  [Vout22] -> Rotor Side/7  "Vout"
  [vqr_ref22] -> Rotor Side/6  "vqr_ref"
  [idr_ref22] -> Mux3/1  "idr_ref"
  Mux3/1 -> Rotor Side/5
  Mux2/1 -> Rotor Side/4
  [Tr_ref22] -> Mux2/1  "Tr_ref_MPPT"
  Mux1/1 -> Rotor Side/2
  [iqr22] -> Mux1/2  "iqr"
  [Tm22] -> Mux2/3  "Tm"

== system Complete_Model/Equivalent Wind Park/300MW Eq WT2/Aerodynamic Model ==
  note: Gearbox ratio
  note: Motor convention : Generated power
      |  is negative
  note: \Omega_m blades
  note: Cp
  note: \lambda = \Omega_m R / v_{wind}
  note: C_p (\lambda)
  note: v_{wind}
  Vw : Inport
  C_p (\lambda) : Lookup_n-D  NumberOfTableDimensions=1  BreakpointsForDimension1=tab_lambda  Table=Cp  InternalRulePriority=Speed  RndMeth=Simplest
  Constant : Constant  Value=Radius
  Divide : Product  Inputs=*/
  Divide1 : Product  Inputs=**/
  Fcn2 : Fcn  Expr=0.5*rho*pi*Radius^2*u(2)^3*u(1)
  Gain : Gain  Gain=1/N
  Gain2 : Gain  Gain=n_wt_agg
  Gain4 : Gain  Gain=-1
  Mux2 : Mux  Inputs=2
  Tm : Outport
  tag scope: P_m6=global, Tm6=global, lambda6=global
  wires:
  Gain4/1 -> Divide/1
  Constant/1 -> Divide1/1
  Divide/1 -> Tm/1, [Tm6]
  [omega_m6] -> Divide/2
  Gain2/1 -> Gain4/1, [P_m6]
  Fcn2/1 -> Gain2/1
  Mux2/1 -> Fcn2/1
  C_p (\lambda)/1 -> Mux2/1
  Divide1/1 -> [lambda6], C_p (\lambda)/1  "lambda"
  Vw/1 -> Mux2/2, Divide1/3
  Gain/1 -> Divide1/2
  [omega_m6] -> Gain/1

== system Complete_Model/Equivalent Wind Park/300MW Eq WT2/Grid Side Control ==
  Vbus_ref : Inport
  Qg_ref : Inport  Port=2
  Vbus : Inport  Port=3
  Ig_mes : Inport  Port=4
  Vs_mes : Inport  Port=5
  Demux : Demux  Outputs=2
  Demux1 : Demux  Outputs=2
  Gain : Gain  Gain=Kqg
  Gain1 : Gain  Gain=Kpg
  Normalized for PWM : Gain  Gain=1/(Vbus/2)
  "PI " : Subsystem
  PI id : Subsystem
  PI iq : Subsystem
  Sum : Sum  Inputs=|++
  Vs_mes1 : InportShadow  Port=5
  abc->alpha/beta : Subsystem
  abc->alpha/beta1 : Subsystem
  alpha/beta->abc : Subsystem
  alpha/beta->d/q : Subsystem
  alpha/beta->d/q1 : Subsystem
  angle2 : Subsystem
  cancellation of coupling terms : Subsystem
  d/q->alpha/beta : Subsystem
  Vabc_ref : Outport
  tag scope: Qg_ref6=global, Vbus_ref6=global, idg6=global, idg_ref6=global, iqg6=global, iqg_ref6=global, vdg6=global, vqg6=global
  wires:
  Vs_mes1/1 -> Sum/2
  angle2/1(theta_g) -> [theta_grid6]
  [theta_grid6] -> alpha/beta->d/q1/2(theta)
  [theta_grid6] -> d/q->alpha/beta/2(theta)
  [theta_grid6] -> alpha/beta->d/q/2(theta)
  Demux/2 -> PI iq/2(meas), [iqg6]
  Demux/1 -> PI id/2(meas), [idg6]
  Vbus/1 -> "PI "/2(meas)
  Demux1/1 -> [vdg6]
  Demux1/2 -> [vqg6]
  alpha/beta->d/q1/1(d/q) -> Demux1/1
  abc->alpha/beta1/1(alpha/beta) -> alpha/beta->d/q1/1(D/Q)
  [Vg6] -> abc->alpha/beta1/1(abc)
  cancellation of coupling terms/1(out) -> d/q->alpha/beta/1(d/q)
  Sum/1 -> Normalized for PWM/1
  alpha/beta->abc/1(abc) -> Sum/1
  Qg_ref/1 -> Gain/1, [Qg_ref6]
  Gain1/1 -> PI id/1(ref), [idg_ref6]  "idg"
  "PI "/1(out) -> Gain1/1  "Pg_ref"
  Gain/1 -> [iqg_ref6], PI iq/1(ref)  "iqg"
  Vbus_ref/1 -> "PI "/1(ref), [Vbus_ref6]
  alpha/beta->d/q/1(d/q) -> Demux/1
  PI iq/1(out) -> cancellation of coupling terms/2(Vq*)
  PI id/1(out) -> cancellation of coupling terms/1(Vd*)
  Vs_mes/1 -> angle2/1(Vs_mes)
  Normalized for PWM/1 -> Vabc_ref/1
  abc->alpha/beta/1(alpha/beta) -> alpha/beta->d/q/1(D/Q)
  Ig_mes/1 -> abc->alpha/beta/1(abc)
  d/q->alpha/beta/1(D/Q) -> alpha/beta->abc/1(D/Q)

== system Complete_Model/Equivalent Wind Park/300MW Eq WT2/Power Calculations ==
  Demux{,1..3} (4 alike) : Demux  Outputs=2
  Divide : Product  Inputs=**
  Gain : Gain  Gain=3/2
  Gain1 : Gain  Gain=-1
  Gain2 : Gain  Gain=-1
  Power (3ph, Instantaneous){1..4} (4 alike) : spsPower3phInstantaneousLib/Power (3ph, Instantaneous)
  Scope{1..8,10..13} (12 alike) : Scope
  Scope9 : Scope  NumInputPorts=2
  "Second-Order " : spsSecondOrderFilterLib/Second-Order Filter  FilterType=Lowpass  Fo=17  Zeta=0.7  Ts=7.4074e-05  Initialize=off  Vac_Init="[0,  0,  60]"  Vdc_Init=0  PlotResponse=off  FreqRange=[0, 500, 1]
      settings: AttributesFormatString=f0=%<Fo>
  Second-Order 1 : spsSecondOrderFilterLib/Second-Order Filter  FilterType=Lowpass  Fo=17  Zeta=0.7  Ts=7.4074e-05  Initialize=off  Vac_Init="[0,  0,  60]"  Vdc_Init=0  PlotResponse=off  FreqRange=[0, 500, 1]
      settings: AttributesFormatString=f0=%<Fo>
  Second-Order Low-Pass Filter {1..7} (7 alike) : spsSecondOrderFilterLib/Second-Order Filter  FilterType=Lowpass  Fo=17  Zeta=0.7  Ts=7.4074e-05  Initialize=off  Vac_Init="[0,  0,  60]"  Vdc_Init=0  PlotResponse=off  FreqRange=[0, 500, 1]
      settings: AttributesFormatString=f0=%<Fo>
  Subsystem : Subsystem
  Subsystem1 : Subsystem
  Subsystem2 : Subsystem
  Subsystem3 : Subsystem
  To Workspace1 : ToWorkspace  VariableName=Iout_d6  SaveFormat=Timeseries  SampleTime=-1
  To Workspace2 : ToWorkspace  VariableName=Ig_d6  SaveFormat=Timeseries  SampleTime=-1
  To Workspace21 : ToWorkspace  VariableName=Vout_d6  SaveFormat=Timeseries  SampleTime=-1
  To Workspace3 : ToWorkspace  VariableName=Vg_d6  SaveFormat=Timeseries  SampleTime=-1
  alpha/beta->d/q : Subsystem
  alpha/beta->d/q1 : Subsystem
  alpha/beta->d/q2 : Subsystem
  alpha/beta->d/q3 : Subsystem
  angle1 : Subsystem
  angle2 : Subsystem
  tag scope: Pg_mes6=global, Pout6=global, Pr_mes_machine6=global, Ps_mes_machine6=global, Qg_mes6=global, Qout6=global, Qr_mes_machine6=global, Qs_mes_machine6=global
  wires:
  [Vg6] -> angle1/1(Vs_mes), Subsystem2/1(abc)
  Subsystem2/1(alpha/beta) -> alpha/beta->d/q2/1(D/Q)
  alpha/beta->d/q2/1(d/q) -> Demux2/1
  Demux2/1 -> To Workspace3/1, Scope10/1
  Demux2/2 -> Scope11/1
  Demux3/2 -> Scope13/1
  Demux3/1 -> Scope12/1, To Workspace2/1
  alpha/beta->d/q3/1(d/q) -> Demux3/1
  Subsystem3/1(alpha/beta) -> alpha/beta->d/q3/1(D/Q)
  angle1/1(theta_g) -> alpha/beta->d/q3/2(theta), alpha/beta->d/q2/2(theta)
  [Ig6] -> Subsystem3/1(abc)
  Gain2/1 -> Second-Order 1/1
  Gain/1 -> Gain2/1
  Gain1/1 -> Scope9/2
  Second-Order 1/1 -> Scope9/1  "Pout"
  Divide/1 -> Gain/1
  [Iout6] -> Subsystem1/1(abc)
  angle2/1(theta_g) -> alpha/beta->d/q/2(theta), alpha/beta->d/q1/2(theta)
  Subsystem1/1(alpha/beta) -> alpha/beta->d/q1/1(D/Q)
  alpha/beta->d/q1/1(d/q) -> Demux1/1
  Demux1/1 -> To Workspace1/1, Scope7/1, Divide/2
  Demux1/2 -> Scope8/1
  Demux/2 -> Scope6/1
  Demux/1 -> Scope2/1, To Workspace21/1, Divide/1
  alpha/beta->d/q/1(d/q) -> Demux/1
  Subsystem/1(alpha/beta) -> alpha/beta->d/q/1(D/Q)
  [Vout6] -> Subsystem/1(abc), angle2/1(Vs_mes)
  [Vr_mes6] -> "Power (3ph, Instantaneous)4"/1
  "Power (3ph, Instantaneous)1"/1 -> Second-Order Low-Pass Filter 7/1  "Pr"
  "Power (3ph, Instantaneous)4"/1 -> Second-Order Low-Pass Filter 5/1  "Pr"
  [Vout6] -> "Power (3ph, Instantaneous)2"/1
  "Power (3ph, Instantaneous)3"/2 -> Second-Order Low-Pass Filter 2/1
  [Vg6] -> "Power (3ph, Instantaneous)1"/1
  Second-Order Low-Pass Filter 2/1 -> [Qs_mes_machine6]  "Q_stator"
  [Vs_mes_machine6] -> "Power (3ph, Instantaneous)3"/1
  "Power (3ph, Instantaneous)2"/1 -> "Second-Order "/1
  Second-Order Low-Pass Filter 5/1 -> [Pr_mes_machine6]  "P_rotor"
  [Is_mes_machine6] -> "Power (3ph, Instantaneous)3"/2, Scope4/1
  Second-Order Low-Pass Filter 7/1 -> [Pg_mes6]  "P_rotor"
  [Ir_mes6] -> "Power (3ph, Instantaneous)4"/2, Scope5/1
  "Second-Order "/1 -> [Pout6], Gain1/1  "Pout"
  Second-Order Low-Pass Filter 6/1 -> [Qg_mes6]  "Q_rotor"
  Second-Order Low-Pass Filter 4/1 -> [Qr_mes_machine6]  "Q_rotor"
  "Power (3ph, Instantaneous)3"/1 -> Second-Order Low-Pass Filter 3/1  "Ps"
  [Ig6] -> Scope1/1, "Power (3ph, Instantaneous)1"/2
  "Power (3ph, Instantaneous)4"/2 -> Second-Order Low-Pass Filter 4/1
  [Iout6] -> "Power (3ph, Instantaneous)2"/2, Scope3/1
  "Power (3ph, Instantaneous)1"/2 -> Second-Order Low-Pass Filter 6/1
  Second-Order Low-Pass Filter 3/1 -> [Ps_mes_machine6]  "P_stator"
  Second-Order Low-Pass Filter 1/1 -> [Qout6]  "Qout"
  "Power (3ph, Instantaneous)2"/2 -> Second-Order Low-Pass Filter 1/1

== system Complete_Model/Equivalent Wind Park/300MW Eq WT2/Rotor Side Control ==
  note: Stator referred
  idr_ref : Inport
  Ir_mes : Inport  Port=2
  Vs_mes : Inport  Port=3
  theta_m_mes : Inport  Port=4
  speed_mes : Inport  Port=5
  D/Q->abc : Subsystem
  D/Q->d/q : Subsystem
  Demux : Demux  Outputs=2
  Gain : Gain  Gain=1/(-1.5*p*(Lm/Ls)*Fs)
  Gain1 : Gain  Gain=1/u
  Gain2 : Gain  Gain=1/u
  MPPT : Subsystem
  Normalized for PWM : Gain  Gain=1/(Vbus/2)
  PI id : Subsystem
  PI iq : Subsystem
  abc->alpha/beta : Subsystem
  angle : Subsystem
  cancellation of coupling terms : Subsystem
  d/q->D/Q : Subsystem
  Vabc_ref : Outport
  tag scope: Tr_ref6=global, idr6=global, idr_ref6=global, iqr6=global, iqr_ref6=global, vrabc_ref6=global
  wires:
  MPPT/1(Tm,opt) -> Gain/1, [Tr_ref6]
  d/q->D/Q/1(D/Q) -> D/Q->abc/1(D/Q)
  abc->alpha/beta/1(alpha/beta) -> D/Q->d/q/1(D/Q)
  Gain2/1 -> [vrabc_ref6], Normalized for PWM/1  "Rotor referred"
  D/Q->abc/1(abc) -> Gain2/1
  Normalized for PWM/1 -> Vabc_ref/1
  Gain1/1 -> abc->alpha/beta/1(abc)
  idr_ref/1 -> PI id/1(ref), [idr_ref6]
  Gain/1 -> PI iq/1(ref), [iqr_ref6]
  Demux/2 -> PI iq/2(meas), [iqr6]
  Demux/1 -> [idr6], PI id/2(meas)
  D/Q->d/q/1(d/q) -> Demux/1  "i_dq = rotor ref frame"
  speed_mes/1 -> MPPT/1(omega_m), cancellation of coupling terms/3(omega_m)
  cancellation of coupling terms/1(out) -> d/q->D/Q/1(d/q)  "Vdr_ref,Vqr_ref"
  PI iq/1(out) -> cancellation of coupling terms/2(Vq*)
  PI id/1(out) -> cancellation of coupling terms/1(Vd*)
  angle/1(theta_r) -> D/Q->d/q/2(theta), d/q->D/Q/2(theta)
  Vs_mes/1 -> angle/1(Vs_mes)
  theta_m_mes/1 -> angle/2(theta_m)
  Ir_mes/1 -> Gain1/1

== system Complete_Model/Equivalent Wind Park/300MW Eq WT2/Subsystem ==
  In1 : Inport
  Bus Selector : BusSelector  OutputSignals=Mechanical.Rotor angle thetam (rad),Mechanical.Rotor speed (wm),Mechanical.Electromagnetic torque Te (N*m)
  Second-Order Low-Pass Filter 3 : spsSecondOrderFilterLib/Second-Order Filter  FilterType=Lowpass  Fo=17  Zeta=0.7  Ts=7.4074e-05  Initialize=off  Vac_Init="[0,  0,  60]"  Vdc_Init=0  PlotResponse=off  FreqRange=[0, 500, 1]
      settings: AttributesFormatString=f0=%<Fo>
  tag scope: Tr6=global, omega_m6=global, theta6=global
  wires:
  Second-Order Low-Pass Filter 3/1 -> [omega_m6]
  Bus Selector/3 -> [Tr6]  "<Electromagnetic torque Te (N*m)>"
  Bus Selector/1 -> [theta6]  "<Rotor angle thetam (rad)>"
  In1/1 -> Bus Selector/1
  Bus Selector/2 -> Second-Order Low-Pass Filter 3/1  "<Rotor speed (wm)>"

== system Complete_Model/Equivalent Wind Park/300MW Eq WT2/Subsystem1 ==
  Constant4 : Constant  Value=0
  Step : Step  Time=50  After=-0.2*Ps  SampleTime=0
  Sum14 : Sum  Inputs=++|
  Out1 : Outport
  wires:
  Sum14/1 -> Out1/1
  Step/1 -> Sum14/1
  Constant4/1 -> Sum14/2

== system Complete_Model/Equivalent Wind Park/300MW Eq WT2/Subsystem2 ==
  Constant1 : Constant  Value=9
  Ramp1 : simulink/Sources/Ramp  slope=-0.4  start=13  InitialOutput=0
  Ramp2 : simulink/Sources/Ramp  slope=0.4  start=8  InitialOutput=0
  Sum1 : Sum  Inputs=++|
  Sum2 : Sum  Inputs=++|
  v_wind : Outport
  tag scope: v_wind6=global
  wires:
  Constant1/1 -> Sum2/2
  Ramp2/1 -> Sum1/1
  Ramp1/1 -> Sum1/2
  Sum2/1 -> [v_wind6], v_wind/1
  Sum1/1 -> Sum2/1

== system Complete_Model/Equivalent Wind Park/300MW Eq WT2/Subsystem8 ==
  Constant4 : Constant  Value=0
  Step : Step  Time=55  After=Idr_rated*0.2  SampleTime=0
  Sum14 : Sum  Inputs=++|
  Out1 : Outport
  wires:
  Sum14/1 -> Out1/1
  Constant4/1 -> Sum14/2
  Step/1 -> Sum14/1
